simulink model "LDW_Real_Life_road_construction_cs"  (Simulink 7.6)
settings: Solver=ode5  SolverMode=SingleTasking  StartTime=0.0  StopTime=inf  FixedStep=1/100  RelTol=1e-3
PreLoadFcn:
  | check_NUTS_defined;
  | 
  | assignin('base',['model_closed_' bdroot],false);
  | 
  | if exist('LDW_mask_sfun.mexw64')==3
  | delete LDW_mask_sfun.mexw64;
  | end
  | 
  | if exist('LDW_mask_sfun.mexw32') ==3
  | delete LDW_mask_sfun.mexw32;
  | end
  | 
  | switch version('-release')
  | case {'2010a', '2010b', '2011a'}
  | addpath('../includes_LKAS/includes_LKAS_2010a');
  | case {'2011b', '2012a', '2012b'}
  | addpath('../includes_LKAS/includes_LKAS_2011b');
  | otherwise
  | addpath('../includes_LKAS/includes_LKAS_2011b');
  | end
InitFcn:
  | PreScanExperimentInitFcn(bdroot)
StopFcn:
  | PreScanExperimentStopFcn(bdroot)
undefined symbols (not defined in the file): DiscIntNeverNeededParam, InitImageOutputDir, x_ini_VRML, y_ini_VRML, z_ini_VRML
libraries: PreScanSimulinkLibrary x69, simulink x26, PreScanUsefulBlocks x25, simulink_extras x15, dspsnks4 x2, simulink_need_slupdate x1
(part 9 of 26)

== system LDW_Real_Life_road_construction_cs/VISUMatrixSignLights_HighwayOverhang_5 ==
  MatrixSignLights : Inport
  SubMuxVisuMatrixSignLights : Demux  Outputs=16
  conv_Orange_1 : Subsystem
  conv_Orange_10 : Subsystem
  conv_Orange_11 : Subsystem
  conv_Orange_12 : Subsystem
  conv_Orange_13 : Subsystem
  conv_Orange_14 : Subsystem
  conv_Orange_15 : Subsystem
  conv_Orange_16 : Subsystem
  conv_Orange_2 : Subsystem
  conv_Orange_3 : Subsystem
  conv_Orange_4 : Subsystem
  conv_Orange_5 : Subsystem
  conv_Orange_6 : Subsystem
  conv_Orange_7 : Subsystem
  conv_Orange_8 : Subsystem
  conv_Orange_9 : Subsystem
  visu_MatrixSignLights_1 : Subsystem  mask(ToSensorWorld){PortName=MatrixSign_17_Light_1, PortIndex=1865, PortWidth=16, TimeStep=1/100}
      mask prompts: PortName "Port Name [-]"; PortIndex "Index [-]"; PortWidth "Port Dimensions [-]"; TimeStep "Upload Period [s]"
      mask help: Sends signal(s) from Simulink to the Sensor World
      mask Help:
      | Sends signal(s) from Simulink to the Sensor World.
      | 
      | - Port Name [-]: specifies the name of the output terminal of the Simulink block.
      | - Index [-]: specifies the start position in the output vector sent to the Sensor World
      | - Port dimensions [-]: specifies the width (number of scalars) of the output port
      | - Upload period [s]: specifies the upload period (in seconds) of the port.
      mask Display: port_label('input', 1, PortName)
  visu_MatrixSignLights_10 : Subsystem  mask(ToSensorWorld){PortName=MatrixSign_19_Light_2, PortIndex=2009, PortWidth=16, TimeStep=1/100}
      mask prompts: PortName "Port Name [-]"; PortIndex "Index [-]"; PortWidth "Port Dimensions [-]"; TimeStep "Upload Period [s]"
      mask help: Sends signal(s) from Simulink to the Sensor World
      mask Help:
      | Sends signal(s) from Simulink to the Sensor World.
      | 
      | - Port Name [-]: specifies the name of the output terminal of the Simulink block.
      | - Index [-]: specifies the start position in the output vector sent to the Sensor World
      | - Port dimensions [-]: specifies the width (number of scalars) of the output port
      | - Upload period [s]: specifies the upload period (in seconds) of the port.
      mask Display: port_label('input', 1, PortName)
  visu_MatrixSignLights_11 : Subsystem  mask(ToSensorWorld){PortName=MatrixSign_19_Light_3, PortIndex=2025, PortWidth=16, TimeStep=1/100}
      mask prompts: PortName "Port Name [-]"; PortIndex "Index [-]"; PortWidth "Port Dimensions [-]"; TimeStep "Upload Period [s]"
      mask help: Sends signal(s) from Simulink to the Sensor World
      mask Help:
      | Sends signal(s) from Simulink to the Sensor World.
      | 
      | - Port Name [-]: specifies the name of the output terminal of the Simulink block.
      | - Index [-]: specifies the start position in the output vector sent to the Sensor World
      | - Port dimensions [-]: specifies the width (number of scalars) of the output port
      | - Upload period [s]: specifies the upload period (in seconds) of the port.
      mask Display: port_label('input', 1, PortName)
  visu_MatrixSignLights_12 : Subsystem  mask(ToSensorWorld){PortName=MatrixSign_19_Light_4, PortIndex=2041, PortWidth=16, TimeStep=1/100}
      mask prompts: PortName "Port Name [-]"; PortIndex "Index [-]"; PortWidth "Port Dimensions [-]"; TimeStep "Upload Period [s]"
      mask help: Sends signal(s) from Simulink to the Sensor World
      mask Help:
      | Sends signal(s) from Simulink to the Sensor World.
      | 
      | - Port Name [-]: specifies the name of the output terminal of the Simulink block.
      | - Index [-]: specifies the start position in the output vector sent to the Sensor World
      | - Port dimensions [-]: specifies the width (number of scalars) of the output port
      | - Upload period [s]: specifies the upload period (in seconds) of the port.
      mask Display: port_label('input', 1, PortName)
  visu_MatrixSignLights_13 : Subsystem  mask(ToSensorWorld){PortName=MatrixSign_20_Light_1, PortIndex=2057, PortWidth=16, TimeStep=1/100}
      mask prompts: PortName "Port Name [-]"; PortIndex "Index [-]"; PortWidth "Port Dimensions [-]"; TimeStep "Upload Period [s]"
      mask help: Sends signal(s) from Simulink to the Sensor World
      mask Help:
      | Sends signal(s) from Simulink to the Sensor World.
      | 
      | - Port Name [-]: specifies the name of the output terminal of the Simulink block.
      | - Index [-]: specifies the start position in the output vector sent to the Sensor World
      | - Port dimensions [-]: specifies the width (number of scalars) of the output port
      | - Upload period [s]: specifies the upload period (in seconds) of the port.
      mask Display: port_label('input', 1, PortName)
  visu_MatrixSignLights_14 : Subsystem  mask(ToSensorWorld){PortName=MatrixSign_20_Light_2, PortIndex=2073, PortWidth=16, TimeStep=1/100}
      mask prompts: PortName "Port Name [-]"; PortIndex "Index [-]"; PortWidth "Port Dimensions [-]"; TimeStep "Upload Period [s]"
      mask help: Sends signal(s) from Simulink to the Sensor World
      mask Help:
      | Sends signal(s) from Simulink to the Sensor World.
      | 
      | - Port Name [-]: specifies the name of the output terminal of the Simulink block.
      | - Index [-]: specifies the start position in the output vector sent to the Sensor World
      | - Port dimensions [-]: specifies the width (number of scalars) of the output port
      | - Upload period [s]: specifies the upload period (in seconds) of the port.
      mask Display: port_label('input', 1, PortName)
  visu_MatrixSignLights_15 : Subsystem  mask(ToSensorWorld){PortName=MatrixSign_20_Light_3, PortIndex=2089, PortWidth=16, TimeStep=1/100}
      mask prompts: PortName "Port Name [-]"; PortIndex "Index [-]"; PortWidth "Port Dimensions [-]"; TimeStep "Upload Period [s]"
      mask help: Sends signal(s) from Simulink to the Sensor World
      mask Help:
      | Sends signal(s) from Simulink to the Sensor World.
      | 
      | - Port Name [-]: specifies the name of the output terminal of the Simulink block.
      | - Index [-]: specifies the start position in the output vector sent to the Sensor World
      | - Port dimensions [-]: specifies the width (number of scalars) of the output port
      | - Upload period [s]: specifies the upload period (in seconds) of the port.
      mask Display: port_label('input', 1, PortName)
  visu_MatrixSignLights_16 : Subsystem  mask(ToSensorWorld){PortName=MatrixSign_20_Light_4, PortIndex=2105, PortWidth=16, TimeStep=1/100}
      mask prompts: PortName "Port Name [-]"; PortIndex "Index [-]"; PortWidth "Port Dimensions [-]"; TimeStep "Upload Period [s]"
      mask help: Sends signal(s) from Simulink to the Sensor World
      mask Help:
      | Sends signal(s) from Simulink to the Sensor World.
      | 
      | - Port Name [-]: specifies the name of the output terminal of the Simulink block.
      | - Index [-]: specifies the start position in the output vector sent to the Sensor World
      | - Port dimensions [-]: specifies the width (number of scalars) of the output port
      | - Upload period [s]: specifies the upload period (in seconds) of the port.
      mask Display: port_label('input', 1, PortName)
  visu_MatrixSignLights_2 : Subsystem  mask(ToSensorWorld){PortName=MatrixSign_17_Light_2, PortIndex=1881, PortWidth=16, TimeStep=1/100}
      mask prompts: PortName "Port Name [-]"; PortIndex "Index [-]"; PortWidth "Port Dimensions [-]"; TimeStep "Upload Period [s]"
      mask help: Sends signal(s) from Simulink to the Sensor World
      mask Help:
      | Sends signal(s) from Simulink to the Sensor World.
      | 
      | - Port Name [-]: specifies the name of the output terminal of the Simulink block.
      | - Index [-]: specifies the start position in the output vector sent to the Sensor World
      | - Port dimensions [-]: specifies the width (number of scalars) of the output port
      | - Upload period [s]: specifies the upload period (in seconds) of the port.
      mask Display: port_label('input', 1, PortName)
  visu_MatrixSignLights_3 : Subsystem  mask(ToSensorWorld){PortName=MatrixSign_17_Light_3, PortIndex=1897, PortWidth=16, TimeStep=1/100}
      mask prompts: PortName "Port Name [-]"; PortIndex "Index [-]"; PortWidth "Port Dimensions [-]"; TimeStep "Upload Period [s]"
      mask help: Sends signal(s) from Simulink to the Sensor World
      mask Help:
      | Sends signal(s) from Simulink to the Sensor World.
      | 
      | - Port Name [-]: specifies the name of the output terminal of the Simulink block.
      | - Index [-]: specifies the start position in the output vector sent to the Sensor World
      | - Port dimensions [-]: specifies the width (number of scalars) of the output port
      | - Upload period [s]: specifies the upload period (in seconds) of the port.
      mask Display: port_label('input', 1, PortName)
  visu_MatrixSignLights_4 : Subsystem  mask(ToSensorWorld){PortName=MatrixSign_17_Light_4, PortIndex=1913, PortWidth=16, TimeStep=1/100}
      mask prompts: PortName "Port Name [-]"; PortIndex "Index [-]"; PortWidth "Port Dimensions [-]"; TimeStep "Upload Period [s]"
      mask help: Sends signal(s) from Simulink to the Sensor World
      mask Help:
      | Sends signal(s) from Simulink to the Sensor World.
      | 
      | - Port Name [-]: specifies the name of the output terminal of the Simulink block.
      | - Index [-]: specifies the start position in the output vector sent to the Sensor World
      | - Port dimensions [-]: specifies the width (number of scalars) of the output port
      | - Upload period [s]: specifies the upload period (in seconds) of the port.
      mask Display: port_label('input', 1, PortName)
  visu_MatrixSignLights_5 : Subsystem  mask(ToSensorWorld){PortName=MatrixSign_18_Light_1, PortIndex=1929, PortWidth=16, TimeStep=1/100}
      mask prompts: PortName "Port Name [-]"; PortIndex "Index [-]"; PortWidth "Port Dimensions [-]"; TimeStep "Upload Period [s]"
      mask help: Sends signal(s) from Simulink to the Sensor World
      mask Help:
      | Sends signal(s) from Simulink to the Sensor World.
      | 
      | - Port Name [-]: specifies the name of the output terminal of the Simulink block.
      | - Index [-]: specifies the start position in the output vector sent to the Sensor World
      | - Port dimensions [-]: specifies the width (number of scalars) of the output port
      | - Upload period [s]: specifies the upload period (in seconds) of the port.
      mask Display: port_label('input', 1, PortName)
  visu_MatrixSignLights_6 : Subsystem  mask(ToSensorWorld){PortName=MatrixSign_18_Light_2, PortIndex=1945, PortWidth=16, TimeStep=1/100}
      mask prompts: PortName "Port Name [-]"; PortIndex "Index [-]"; PortWidth "Port Dimensions [-]"; TimeStep "Upload Period [s]"
      mask help: Sends signal(s) from Simulink to the Sensor World
      mask Help:
      | Sends signal(s) from Simulink to the Sensor World.
      | 
      | - Port Name [-]: specifies the name of the output terminal of the Simulink block.
      | - Index [-]: specifies the start position in the output vector sent to the Sensor World
      | - Port dimensions [-]: specifies the width (number of scalars) of the output port
      | - Upload period [s]: specifies the upload period (in seconds) of the port.
      mask Display: port_label('input', 1, PortName)
  visu_MatrixSignLights_7 : Subsystem  mask(ToSensorWorld){PortName=MatrixSign_18_Light_3, PortIndex=1961, PortWidth=16, TimeStep=1/100}
      mask prompts: PortName "Port Name [-]"; PortIndex "Index [-]"; PortWidth "Port Dimensions [-]"; TimeStep "Upload Period [s]"
      mask help: Sends signal(s) from Simulink to the Sensor World
      mask Help:
      | Sends signal(s) from Simulink to the Sensor World.
      | 
      | - Port Name [-]: specifies the name of the output terminal of the Simulink block.
      | - Index [-]: specifies the start position in the output vector sent to the Sensor World
      | - Port dimensions [-]: specifies the width (number of scalars) of the output port
      | - Upload period [s]: specifies the upload period (in seconds) of the port.
      mask Display: port_label('input', 1, PortName)
  visu_MatrixSignLights_8 : Subsystem  mask(ToSensorWorld){PortName=MatrixSign_18_Light_4, PortIndex=1977, PortWidth=16, TimeStep=1/100}
      mask prompts: PortName "Port Name [-]"; PortIndex "Index [-]"; PortWidth "Port Dimensions [-]"; TimeStep "Upload Period [s]"
      mask help: Sends signal(s) from Simulink to the Sensor World
      mask Help:
      | Sends signal(s) from Simulink to the Sensor World.
      | 
      | - Port Name [-]: specifies the name of the output terminal of the Simulink block.
      | - Index [-]: specifies the start position in the output vector sent to the Sensor World
      | - Port dimensions [-]: specifies the width (number of scalars) of the output port
      | - Upload period [s]: specifies the upload period (in seconds) of the port.
      mask Display: port_label('input', 1, PortName)
  visu_MatrixSignLights_9 : Subsystem  mask(ToSensorWorld){PortName=MatrixSign_19_Light_1, PortIndex=1993, PortWidth=16, TimeStep=1/100}
      mask prompts: PortName "Port Name [-]"; PortIndex "Index [-]"; PortWidth "Port Dimensions [-]"; TimeStep "Upload Period [s]"
      mask help: Sends signal(s) from Simulink to the Sensor World
      mask Help:
      | Sends signal(s) from Simulink to the Sensor World.
      | 
      | - Port Name [-]: specifies the name of the output terminal of the Simulink block.
      | - Index [-]: specifies the start position in the output vector sent to the Sensor World
      | - Port dimensions [-]: specifies the width (number of scalars) of the output port
      | - Upload period [s]: specifies the upload period (in seconds) of the port.
      mask Display: port_label('input', 1, PortName)
  wires:
  conv_Orange_1/1(RGBcolor) -> visu_MatrixSignLights_1/1(Y)
  MatrixSignLights/1 -> SubMuxVisuMatrixSignLights/1
  SubMuxVisuMatrixSignLights/1 -> conv_Orange_1/1(on_off)
  SubMuxVisuMatrixSignLights/2 -> conv_Orange_2/1(on_off)
  conv_Orange_2/1(RGBcolor) -> visu_MatrixSignLights_2/1(Y)
  SubMuxVisuMatrixSignLights/3 -> conv_Orange_3/1(on_off)
  conv_Orange_3/1(RGBcolor) -> visu_MatrixSignLights_3/1(Y)
  SubMuxVisuMatrixSignLights/4 -> conv_Orange_4/1(on_off)
  conv_Orange_4/1(RGBcolor) -> visu_MatrixSignLights_4/1(Y)
  SubMuxVisuMatrixSignLights/5 -> conv_Orange_5/1(on_off)
  conv_Orange_5/1(RGBcolor) -> visu_MatrixSignLights_5/1(Y)
  SubMuxVisuMatrixSignLights/6 -> conv_Orange_6/1(on_off)
  conv_Orange_6/1(RGBcolor) -> visu_MatrixSignLights_6/1(Y)
  SubMuxVisuMatrixSignLights/7 -> conv_Orange_7/1(on_off)
  conv_Orange_7/1(RGBcolor) -> visu_MatrixSignLights_7/1(Y)
  SubMuxVisuMatrixSignLights/8 -> conv_Orange_8/1(on_off)
  conv_Orange_8/1(RGBcolor) -> visu_MatrixSignLights_8/1(Y)
  SubMuxVisuMatrixSignLights/9 -> conv_Orange_9/1(on_off)
  conv_Orange_9/1(RGBcolor) -> visu_MatrixSignLights_9/1(Y)
  SubMuxVisuMatrixSignLights/10 -> conv_Orange_10/1(on_off)
  conv_Orange_10/1(RGBcolor) -> visu_MatrixSignLights_10/1(Y)
  SubMuxVisuMatrixSignLights/11 -> conv_Orange_11/1(on_off)
  conv_Orange_11/1(RGBcolor) -> visu_MatrixSignLights_11/1(Y)
  SubMuxVisuMatrixSignLights/12 -> conv_Orange_12/1(on_off)
  conv_Orange_12/1(RGBcolor) -> visu_MatrixSignLights_12/1(Y)
  SubMuxVisuMatrixSignLights/13 -> conv_Orange_13/1(on_off)
  conv_Orange_13/1(RGBcolor) -> visu_MatrixSignLights_13/1(Y)
  SubMuxVisuMatrixSignLights/14 -> conv_Orange_14/1(on_off)
  conv_Orange_14/1(RGBcolor) -> visu_MatrixSignLights_14/1(Y)
  SubMuxVisuMatrixSignLights/15 -> conv_Orange_15/1(on_off)
  conv_Orange_15/1(RGBcolor) -> visu_MatrixSignLights_15/1(Y)
  SubMuxVisuMatrixSignLights/16 -> conv_Orange_16/1(on_off)
  conv_Orange_16/1(RGBcolor) -> visu_MatrixSignLights_16/1(Y)

== system LDW_Real_Life_road_construction_cs/VISUMatrixSignLights_HighwayOverhang_6 ==
  MatrixSignLights : Inport
  SubMuxVisuMatrixSignLights : Demux  Outputs=16
  conv_Orange_1 : Subsystem
  conv_Orange_10 : Subsystem
  conv_Orange_11 : Subsystem
  conv_Orange_12 : Subsystem
  conv_Orange_13 : Subsystem
  conv_Orange_14 : Subsystem
  conv_Orange_15 : Subsystem
  conv_Orange_16 : Subsystem
  conv_Orange_2 : Subsystem
  conv_Orange_3 : Subsystem
  conv_Orange_4 : Subsystem
  conv_Orange_5 : Subsystem
  conv_Orange_6 : Subsystem
  conv_Orange_7 : Subsystem
  conv_Orange_8 : Subsystem
  conv_Orange_9 : Subsystem
  visu_MatrixSignLights_1 : Subsystem  mask(ToSensorWorld){PortName=MatrixSign_21_Light_1, PortIndex=2125, PortWidth=16, TimeStep=1/100}
      mask prompts: PortName "Port Name [-]"; PortIndex "Index [-]"; PortWidth "Port Dimensions [-]"; TimeStep "Upload Period [s]"
      mask help: Sends signal(s) from Simulink to the Sensor World
      mask Help:
      | Sends signal(s) from Simulink to the Sensor World.
      | 
      | - Port Name [-]: specifies the name of the output terminal of the Simulink block.
      | - Index [-]: specifies the start position in the output vector sent to the Sensor World
      | - Port dimensions [-]: specifies the width (number of scalars) of the output port
      | - Upload period [s]: specifies the upload period (in seconds) of the port.
      mask Display: port_label('input', 1, PortName)
  visu_MatrixSignLights_10 : Subsystem  mask(ToSensorWorld){PortName=MatrixSign_23_Light_2, PortIndex=2269, PortWidth=16, TimeStep=1/100}
      mask prompts: PortName "Port Name [-]"; PortIndex "Index [-]"; PortWidth "Port Dimensions [-]"; TimeStep "Upload Period [s]"
      mask help: Sends signal(s) from Simulink to the Sensor World
      mask Help:
      | Sends signal(s) from Simulink to the Sensor World.
      | 
      | - Port Name [-]: specifies the name of the output terminal of the Simulink block.
      | - Index [-]: specifies the start position in the output vector sent to the Sensor World
      | - Port dimensions [-]: specifies the width (number of scalars) of the output port
      | - Upload period [s]: specifies the upload period (in seconds) of the port.
      mask Display: port_label('input', 1, PortName)
  visu_MatrixSignLights_11 : Subsystem  mask(ToSensorWorld){PortName=MatrixSign_23_Light_3, PortIndex=2285, PortWidth=16, TimeStep=1/100}
      mask prompts: PortName "Port Name [-]"; PortIndex "Index [-]"; PortWidth "Port Dimensions [-]"; TimeStep "Upload Period [s]"
      mask help: Sends signal(s) from Simulink to the Sensor World
      mask Help:
      | Sends signal(s) from Simulink to the Sensor World.
      | 
      | - Port Name [-]: specifies the name of the output terminal of the Simulink block.
      | - Index [-]: specifies the start position in the output vector sent to the Sensor World
      | - Port dimensions [-]: specifies the width (number of scalars) of the output port
      | - Upload period [s]: specifies the upload period (in seconds) of the port.
      mask Display: port_label('input', 1, PortName)
  visu_MatrixSignLights_12 : Subsystem  mask(ToSensorWorld){PortName=MatrixSign_23_Light_4, PortIndex=2301, PortWidth=16, TimeStep=1/100}
      mask prompts: PortName "Port Name [-]"; PortIndex "Index [-]"; PortWidth "Port Dimensions [-]"; TimeStep "Upload Period [s]"
      mask help: Sends signal(s) from Simulink to the Sensor World
      mask Help:
      | Sends signal(s) from Simulink to the Sensor World.
      | 
      | - Port Name [-]: specifies the name of the output terminal of the Simulink block.
      | - Index [-]: specifies the start position in the output vector sent to the Sensor World
      | - Port dimensions [-]: specifies the width (number of scalars) of the output port
      | - Upload period [s]: specifies the upload period (in seconds) of the port.
      mask Display: port_label('input', 1, PortName)
  visu_MatrixSignLights_13 : Subsystem  mask(ToSensorWorld){PortName=MatrixSign_24_Light_1, PortIndex=2317, PortWidth=16, TimeStep=1/100}
      mask prompts: PortName "Port Name [-]"; PortIndex "Index [-]"; PortWidth "Port Dimensions [-]"; TimeStep "Upload Period [s]"
      mask help: Sends signal(s) from Simulink to the Sensor World
      mask Help:
      | Sends signal(s) from Simulink to the Sensor World.
      | 
      | - Port Name [-]: specifies the name of the output terminal of the Simulink block.
      | - Index [-]: specifies the start position in the output vector sent to the Sensor World
      | - Port dimensions [-]: specifies the width (number of scalars) of the output port
      | - Upload period [s]: specifies the upload period (in seconds) of the port.
      mask Display: port_label('input', 1, PortName)
  visu_MatrixSignLights_14 : Subsystem  mask(ToSensorWorld){PortName=MatrixSign_24_Light_2, PortIndex=2333, PortWidth=16, TimeStep=1/100}
      mask prompts: PortName "Port Name [-]"; PortIndex "Index [-]"; PortWidth "Port Dimensions [-]"; TimeStep "Upload Period [s]"
      mask help: Sends signal(s) from Simulink to the Sensor World
      mask Help:
      | Sends signal(s) from Simulink to the Sensor World.
      | 
      | - Port Name [-]: specifies the name of the output terminal of the Simulink block.
      | - Index [-]: specifies the start position in the output vector sent to the Sensor World
      | - Port dimensions [-]: specifies the width (number of scalars) of the output port
      | - Upload period [s]: specifies the upload period (in seconds) of the port.
      mask Display: port_label('input', 1, PortName)
  visu_MatrixSignLights_15 : Subsystem  mask(ToSensorWorld){PortName=MatrixSign_24_Light_3, PortIndex=2349, PortWidth=16, TimeStep=1/100}
      mask prompts: PortName "Port Name [-]"; PortIndex "Index [-]"; PortWidth "Port Dimensions [-]"; TimeStep "Upload Period [s]"
      mask help: Sends signal(s) from Simulink to the Sensor World
      mask Help:
      | Sends signal(s) from Simulink to the Sensor World.
      | 
      | - Port Name [-]: specifies the name of the output terminal of the Simulink block.
      | - Index [-]: specifies the start position in the output vector sent to the Sensor World
      | - Port dimensions [-]: specifies the width (number of scalars) of the output port
      | - Upload period [s]: specifies the upload period (in seconds) of the port.
      mask Display: port_label('input', 1, PortName)
  visu_MatrixSignLights_16 : Subsystem  mask(ToSensorWorld){PortName=MatrixSign_24_Light_4, PortIndex=2365, PortWidth=16, TimeStep=1/100}
      mask prompts: PortName "Port Name [-]"; PortIndex "Index [-]"; PortWidth "Port Dimensions [-]"; TimeStep "Upload Period [s]"
      mask help: Sends signal(s) from Simulink to the Sensor World
      mask Help:
      | Sends signal(s) from Simulink to the Sensor World.
      | 
      | - Port Name [-]: specifies the name of the output terminal of the Simulink block.
      | - Index [-]: specifies the start position in the output vector sent to the Sensor World
      | - Port dimensions [-]: specifies the width (number of scalars) of the output port
      | - Upload period [s]: specifies the upload period (in seconds) of the port.
      mask Display: port_label('input', 1, PortName)
  visu_MatrixSignLights_2 : Subsystem  mask(ToSensorWorld){PortName=MatrixSign_21_Light_2, PortIndex=2141, PortWidth=16, TimeStep=1/100}
      mask prompts: PortName "Port Name [-]"; PortIndex "Index [-]"; PortWidth "Port Dimensions [-]"; TimeStep "Upload Period [s]"
      mask help: Sends signal(s) from Simulink to the Sensor World
      mask Help:
      | Sends signal(s) from Simulink to the Sensor World.
      | 
      | - Port Name [-]: specifies the name of the output terminal of the Simulink block.
      | - Index [-]: specifies the start position in the output vector sent to the Sensor World
      | - Port dimensions [-]: specifies the width (number of scalars) of the output port
      | - Upload period [s]: specifies the upload period (in seconds) of the port.
      mask Display: port_label('input', 1, PortName)
  visu_MatrixSignLights_3 : Subsystem  mask(ToSensorWorld){PortName=MatrixSign_21_Light_3, PortIndex=2157, PortWidth=16, TimeStep=1/100}
      mask prompts: PortName "Port Name [-]"; PortIndex "Index [-]"; PortWidth "Port Dimensions [-]"; TimeStep "Upload Period [s]"
      mask help: Sends signal(s) from Simulink to the Sensor World
      mask Help:
      | Sends signal(s) from Simulink to the Sensor World.
      | 
      | - Port Name [-]: specifies the name of the output terminal of the Simulink block.
      | - Index [-]: specifies the start position in the output vector sent to the Sensor World
      | - Port dimensions [-]: specifies the width (number of scalars) of the output port
      | - Upload period [s]: specifies the upload period (in seconds) of the port.
      mask Display: port_label('input', 1, PortName)
  visu_MatrixSignLights_4 : Subsystem  mask(ToSensorWorld){PortName=MatrixSign_21_Light_4, PortIndex=2173, PortWidth=16, TimeStep=1/100}
      mask prompts: PortName "Port Name [-]"; PortIndex "Index [-]"; PortWidth "Port Dimensions [-]"; TimeStep "Upload Period [s]"
      mask help: Sends signal(s) from Simulink to the Sensor World
      mask Help:
      | Sends signal(s) from Simulink to the Sensor World.
      | 
      | - Port Name [-]: specifies the name of the output terminal of the Simulink block.
      | - Index [-]: specifies the start position in the output vector sent to the Sensor World
      | - Port dimensions [-]: specifies the width (number of scalars) of the output port
      | - Upload period [s]: specifies the upload period (in seconds) of the port.
      mask Display: port_label('input', 1, PortName)
  visu_MatrixSignLights_5 : Subsystem  mask(ToSensorWorld){PortName=MatrixSign_22_Light_1, PortIndex=2189, PortWidth=16, TimeStep=1/100}
      mask prompts: PortName "Port Name [-]"; PortIndex "Index [-]"; PortWidth "Port Dimensions [-]"; TimeStep "Upload Period [s]"
      mask help: Sends signal(s) from Simulink to the Sensor World
      mask Help:
      | Sends signal(s) from Simulink to the Sensor World.
      | 
      | - Port Name [-]: specifies the name of the output terminal of the Simulink block.
      | - Index [-]: specifies the start position in the output vector sent to the Sensor World
      | - Port dimensions [-]: specifies the width (number of scalars) of the output port
      | - Upload period [s]: specifies the upload period (in seconds) of the port.
      mask Display: port_label('input', 1, PortName)
  visu_MatrixSignLights_6 : Subsystem  mask(ToSensorWorld){PortName=MatrixSign_22_Light_2, PortIndex=2205, PortWidth=16, TimeStep=1/100}
      mask prompts: PortName "Port Name [-]"; PortIndex "Index [-]"; PortWidth "Port Dimensions [-]"; TimeStep "Upload Period [s]"
      mask help: Sends signal(s) from Simulink to the Sensor World
      mask Help:
      | Sends signal(s) from Simulink to the Sensor World.
      | 
      | - Port Name [-]: specifies the name of the output terminal of the Simulink block.
      | - Index [-]: specifies the start position in the output vector sent to the Sensor World
      | - Port dimensions [-]: specifies the width (number of scalars) of the output port
      | - Upload period [s]: specifies the upload period (in seconds) of the port.
      mask Display: port_label('input', 1, PortName)
  visu_MatrixSignLights_7 : Subsystem  mask(ToSensorWorld){PortName=MatrixSign_22_Light_3, PortIndex=2221, PortWidth=16, TimeStep=1/100}
      mask prompts: PortName "Port Name [-]"; PortIndex "Index [-]"; PortWidth "Port Dimensions [-]"; TimeStep "Upload Period [s]"
      mask help: Sends signal(s) from Simulink to the Sensor World
      mask Help:
      | Sends signal(s) from Simulink to the Sensor World.
      | 
      | - Port Name [-]: specifies the name of the output terminal of the Simulink block.
      | - Index [-]: specifies the start position in the output vector sent to the Sensor World
      | - Port dimensions [-]: specifies the width (number of scalars) of the output port
      | - Upload period [s]: specifies the upload period (in seconds) of the port.
      mask Display: port_label('input', 1, PortName)
  visu_MatrixSignLights_8 : Subsystem  mask(ToSensorWorld){PortName=MatrixSign_22_Light_4, PortIndex=2237, PortWidth=16, TimeStep=1/100}
      mask prompts: PortName "Port Name [-]"; PortIndex "Index [-]"; PortWidth "Port Dimensions [-]"; TimeStep "Upload Period [s]"
      mask help: Sends signal(s) from Simulink to the Sensor World
      mask Help:
      | Sends signal(s) from Simulink to the Sensor World.
      | 
      | - Port Name [-]: specifies the name of the output terminal of the Simulink block.
      | - Index [-]: specifies the start position in the output vector sent to the Sensor World
      | - Port dimensions [-]: specifies the width (number of scalars) of the output port
      | - Upload period [s]: specifies the upload period (in seconds) of the port.
      mask Display: port_label('input', 1, PortName)
  visu_MatrixSignLights_9 : Subsystem  mask(ToSensorWorld){PortName=MatrixSign_23_Light_1, PortIndex=2253, PortWidth=16, TimeStep=1/100}
      mask prompts: PortName "Port Name [-]"; PortIndex "Index [-]"; PortWidth "Port Dimensions [-]"; TimeStep "Upload Period [s]"
      mask help: Sends signal(s) from Simulink to the Sensor World
      mask Help:
      | Sends signal(s) from Simulink to the Sensor World.
      | 
      | - Port Name [-]: specifies the name of the output terminal of the Simulink block.
      | - Index [-]: specifies the start position in the output vector sent to the Sensor World
      | - Port dimensions [-]: specifies the width (number of scalars) of the output port
      | - Upload period [s]: specifies the upload period (in seconds) of the port.
      mask Display: port_label('input', 1, PortName)
  wires:
  conv_Orange_1/1(RGBcolor) -> visu_MatrixSignLights_1/1(Y)
  MatrixSignLights/1 -> SubMuxVisuMatrixSignLights/1
  SubMuxVisuMatrixSignLights/1 -> conv_Orange_1/1(on_off)
  SubMuxVisuMatrixSignLights/2 -> conv_Orange_2/1(on_off)
  conv_Orange_2/1(RGBcolor) -> visu_MatrixSignLights_2/1(Y)
  SubMuxVisuMatrixSignLights/3 -> conv_Orange_3/1(on_off)
  conv_Orange_3/1(RGBcolor) -> visu_MatrixSignLights_3/1(Y)
  SubMuxVisuMatrixSignLights/4 -> conv_Orange_4/1(on_off)
  conv_Orange_4/1(RGBcolor) -> visu_MatrixSignLights_4/1(Y)
  SubMuxVisuMatrixSignLights/5 -> conv_Orange_5/1(on_off)
  conv_Orange_5/1(RGBcolor) -> visu_MatrixSignLights_5/1(Y)
  SubMuxVisuMatrixSignLights/6 -> conv_Orange_6/1(on_off)
  conv_Orange_6/1(RGBcolor) -> visu_MatrixSignLights_6/1(Y)
  SubMuxVisuMatrixSignLights/7 -> conv_Orange_7/1(on_off)
  conv_Orange_7/1(RGBcolor) -> visu_MatrixSignLights_7/1(Y)
  SubMuxVisuMatrixSignLights/8 -> conv_Orange_8/1(on_off)
  conv_Orange_8/1(RGBcolor) -> visu_MatrixSignLights_8/1(Y)
  SubMuxVisuMatrixSignLights/9 -> conv_Orange_9/1(on_off)
  conv_Orange_9/1(RGBcolor) -> visu_MatrixSignLights_9/1(Y)
  SubMuxVisuMatrixSignLights/10 -> conv_Orange_10/1(on_off)
  conv_Orange_10/1(RGBcolor) -> visu_MatrixSignLights_10/1(Y)
  SubMuxVisuMatrixSignLights/11 -> conv_Orange_11/1(on_off)
  conv_Orange_11/1(RGBcolor) -> visu_MatrixSignLights_11/1(Y)
  SubMuxVisuMatrixSignLights/12 -> conv_Orange_12/1(on_off)
  conv_Orange_12/1(RGBcolor) -> visu_MatrixSignLights_12/1(Y)
  SubMuxVisuMatrixSignLights/13 -> conv_Orange_13/1(on_off)
  conv_Orange_13/1(RGBcolor) -> visu_MatrixSignLights_13/1(Y)
  SubMuxVisuMatrixSignLights/14 -> conv_Orange_14/1(on_off)
  conv_Orange_14/1(RGBcolor) -> visu_MatrixSignLights_14/1(Y)
  SubMuxVisuMatrixSignLights/15 -> conv_Orange_15/1(on_off)
  conv_Orange_15/1(RGBcolor) -> visu_MatrixSignLights_15/1(Y)
  SubMuxVisuMatrixSignLights/16 -> conv_Orange_16/1(on_off)
  conv_Orange_16/1(RGBcolor) -> visu_MatrixSignLights_16/1(Y)

== system LDW_Real_Life_road_construction_cs/VISUMatrixSign_HighwayOverhang_1 ==
  MatrixSigns : Inport
  SubMuxVisuMatrixSign : Demux
  visu_MatrixSign_1 : Subsystem  mask(ToSensorWorld){PortName=MatrixSign_1, PortIndex=821, PortWidth=1, TimeStep=1/100}
      mask prompts: PortName "Port Name [-]"; PortIndex "Index [-]"; PortWidth "Port Dimensions [-]"; TimeStep "Upload Period [s]"
      mask help: Sends signal(s) from Simulink to the Sensor World
      mask Help:
      | Sends signal(s) from Simulink to the Sensor World.
      | 
      | - Port Name [-]: specifies the name of the output terminal of the Simulink block.
      | - Index [-]: specifies the start position in the output vector sent to the Sensor World
      | - Port dimensions [-]: specifies the width (number of scalars) of the output port
      | - Upload period [s]: specifies the upload period (in seconds) of the port.
      mask Display: port_label('input', 1, PortName)
  visu_MatrixSign_2 : Subsystem  mask(ToSensorWorld){PortName=MatrixSign_2, PortIndex=822, PortWidth=1, TimeStep=1/100}
      mask prompts: PortName "Port Name [-]"; PortIndex "Index [-]"; PortWidth "Port Dimensions [-]"; TimeStep "Upload Period [s]"
      mask help: Sends signal(s) from Simulink to the Sensor World
      mask Help:
      | Sends signal(s) from Simulink to the Sensor World.
      | 
      | - Port Name [-]: specifies the name of the output terminal of the Simulink block.
      | - Index [-]: specifies the start position in the output vector sent to the Sensor World
      | - Port dimensions [-]: specifies the width (number of scalars) of the output port
      | - Upload period [s]: specifies the upload period (in seconds) of the port.
      mask Display: port_label('input', 1, PortName)
  visu_MatrixSign_3 : Subsystem  mask(ToSensorWorld){PortName=MatrixSign_3, PortIndex=823, PortWidth=1, TimeStep=1/100}
      mask prompts: PortName "Port Name [-]"; PortIndex "Index [-]"; PortWidth "Port Dimensions [-]"; TimeStep "Upload Period [s]"
      mask help: Sends signal(s) from Simulink to the Sensor World
      mask Help:
      | Sends signal(s) from Simulink to the Sensor World.
      | 
      | - Port Name [-]: specifies the name of the output terminal of the Simulink block.
      | - Index [-]: specifies the start position in the output vector sent to the Sensor World
      | - Port dimensions [-]: specifies the width (number of scalars) of the output port
      | - Upload period [s]: specifies the upload period (in seconds) of the port.
      mask Display: port_label('input', 1, PortName)
  visu_MatrixSign_4 : Subsystem  mask(ToSensorWorld){PortName=MatrixSign_4, PortIndex=824, PortWidth=1, TimeStep=1/100}
      mask prompts: PortName "Port Name [-]"; PortIndex "Index [-]"; PortWidth "Port Dimensions [-]"; TimeStep "Upload Period [s]"
      mask help: Sends signal(s) from Simulink to the Sensor World
      mask Help:
      | Sends signal(s) from Simulink to the Sensor World.
      | 
      | - Port Name [-]: specifies the name of the output terminal of the Simulink block.
      | - Index [-]: specifies the start position in the output vector sent to the Sensor World
      | - Port dimensions [-]: specifies the width (number of scalars) of the output port
      | - Upload period [s]: specifies the upload period (in seconds) of the port.
      mask Display: port_label('input', 1, PortName)
  wires:
  SubMuxVisuMatrixSign/1 -> visu_MatrixSign_1/1(Y)
  MatrixSigns/1 -> SubMuxVisuMatrixSign/1
  SubMuxVisuMatrixSign/2 -> visu_MatrixSign_2/1(Y)
  SubMuxVisuMatrixSign/3 -> visu_MatrixSign_3/1(Y)
  SubMuxVisuMatrixSign/4 -> visu_MatrixSign_4/1(Y)

== system LDW_Real_Life_road_construction_cs/VISUMatrixSign_HighwayOverhang_2 ==
  MatrixSigns : Inport
  SubMuxVisuMatrixSign : Demux
  visu_MatrixSign_1 : Subsystem  mask(ToSensorWorld){PortName=MatrixSign_5, PortIndex=1081, PortWidth=1, TimeStep=1/100}
      mask prompts: PortName "Port Name [-]"; PortIndex "Index [-]"; PortWidth "Port Dimensions [-]"; TimeStep "Upload Period [s]"
      mask help: Sends signal(s) from Simulink to the Sensor World
      mask Help:
      | Sends signal(s) from Simulink to the Sensor World.
      | 
      | - Port Name [-]: specifies the name of the output terminal of the Simulink block.
      | - Index [-]: specifies the start position in the output vector sent to the Sensor World
      | - Port dimensions [-]: specifies the width (number of scalars) of the output port
      | - Upload period [s]: specifies the upload period (in seconds) of the port.
      mask Display: port_label('input', 1, PortName)
  visu_MatrixSign_2 : Subsystem  mask(ToSensorWorld){PortName=MatrixSign_6, PortIndex=1082, PortWidth=1, TimeStep=1/100}
      mask prompts: PortName "Port Name [-]"; PortIndex "Index [-]"; PortWidth "Port Dimensions [-]"; TimeStep "Upload Period [s]"
      mask help: Sends signal(s) from Simulink to the Sensor World
      mask Help:
      | Sends signal(s) from Simulink to the Sensor World.
      | 
      | - Port Name [-]: specifies the name of the output terminal of the Simulink block.
      | - Index [-]: specifies the start position in the output vector sent to the Sensor World
      | - Port dimensions [-]: specifies the width (number of scalars) of the output port
      | - Upload period [s]: specifies the upload period (in seconds) of the port.
      mask Display: port_label('input', 1, PortName)
  visu_MatrixSign_3 : Subsystem  mask(ToSensorWorld){PortName=MatrixSign_7, PortIndex=1083, PortWidth=1, TimeStep=1/100}
      mask prompts: PortName "Port Name [-]"; PortIndex "Index [-]"; PortWidth "Port Dimensions [-]"; TimeStep "Upload Period [s]"
      mask help: Sends signal(s) from Simulink to the Sensor World
      mask Help:
      | Sends signal(s) from Simulink to the Sensor World.
      | 
      | - Port Name [-]: specifies the name of the output terminal of the Simulink block.
      | - Index [-]: specifies the start position in the output vector sent to the Sensor World
      | - Port dimensions [-]: specifies the width (number of scalars) of the output port
      | - Upload period [s]: specifies the upload period (in seconds) of the port.
      mask Display: port_label('input', 1, PortName)
  visu_MatrixSign_4 : Subsystem  mask(ToSensorWorld){PortName=MatrixSign_8, PortIndex=1084, PortWidth=1, TimeStep=1/100}
      mask prompts: PortName "Port Name [-]"; PortIndex "Index [-]"; PortWidth "Port Dimensions [-]"; TimeStep "Upload Period [s]"
      mask help: Sends signal(s) from Simulink to the Sensor World
      mask Help:
      | Sends signal(s) from Simulink to the Sensor World.
      | 
      | - Port Name [-]: specifies the name of the output terminal of the Simulink block.
      | - Index [-]: specifies the start position in the output vector sent to the Sensor World
      | - Port dimensions [-]: specifies the width (number of scalars) of the output port
      | - Upload period [s]: specifies the upload period (in seconds) of the port.
      mask Display: port_label('input', 1, PortName)
  wires:
  SubMuxVisuMatrixSign/1 -> visu_MatrixSign_1/1(Y)
  MatrixSigns/1 -> SubMuxVisuMatrixSign/1
  SubMuxVisuMatrixSign/2 -> visu_MatrixSign_2/1(Y)
  SubMuxVisuMatrixSign/3 -> visu_MatrixSign_3/1(Y)
  SubMuxVisuMatrixSign/4 -> visu_MatrixSign_4/1(Y)

== system LDW_Real_Life_road_construction_cs/VISUMatrixSign_HighwayOverhang_3 ==
  MatrixSigns : Inport
  SubMuxVisuMatrixSign : Demux
  visu_MatrixSign_1 : Subsystem  mask(ToSensorWorld){PortName=MatrixSign_9, PortIndex=1341, PortWidth=1, TimeStep=1/100}
      mask prompts: PortName "Port Name [-]"; PortIndex "Index [-]"; PortWidth "Port Dimensions [-]"; TimeStep "Upload Period [s]"
      mask help: Sends signal(s) from Simulink to the Sensor World
      mask Help:
      | Sends signal(s) from Simulink to the Sensor World.
      | 
      | - Port Name [-]: specifies the name of the output terminal of the Simulink block.
      | - Index [-]: specifies the start position in the output vector sent to the Sensor World
      | - Port dimensions [-]: specifies the width (number of scalars) of the output port
      | - Upload period [s]: specifies the upload period (in seconds) of the port.
      mask Display: port_label('input', 1, PortName)
  visu_MatrixSign_2 : Subsystem  mask(ToSensorWorld){PortName=MatrixSign_10, PortIndex=1342, PortWidth=1, TimeStep=1/100}
      mask prompts: PortName "Port Name [-]"; PortIndex "Index [-]"; PortWidth "Port Dimensions [-]"; TimeStep "Upload Period [s]"
      mask help: Sends signal(s) from Simulink to the Sensor World
      mask Help:
      | Sends signal(s) from Simulink to the Sensor World.
      | 
      | - Port Name [-]: specifies the name of the output terminal of the Simulink block.
      | - Index [-]: specifies the start position in the output vector sent to the Sensor World
      | - Port dimensions [-]: specifies the width (number of scalars) of the output port
      | - Upload period [s]: specifies the upload period (in seconds) of the port.
      mask Display: port_label('input', 1, PortName)
  visu_MatrixSign_3 : Subsystem  mask(ToSensorWorld){PortName=MatrixSign_11, PortIndex=1343, PortWidth=1, TimeStep=1/100}
      mask prompts: PortName "Port Name [-]"; PortIndex "Index [-]"; PortWidth "Port Dimensions [-]"; TimeStep "Upload Period [s]"
      mask help: Sends signal(s) from Simulink to the Sensor World
      mask Help:
      | Sends signal(s) from Simulink to the Sensor World.
      | 
      | - Port Name [-]: specifies the name of the output terminal of the Simulink block.
      | - Index [-]: specifies the start position in the output vector sent to the Sensor World
      | - Port dimensions [-]: specifies the width (number of scalars) of the output port
      | - Upload period [s]: specifies the upload period (in seconds) of the port.
      mask Display: port_label('input', 1, PortName)
  visu_MatrixSign_4 : Subsystem  mask(ToSensorWorld){PortName=MatrixSign_12, PortIndex=1344, PortWidth=1, TimeStep=1/100}
      mask prompts: PortName "Port Name [-]"; PortIndex "Index [-]"; PortWidth "Port Dimensions [-]"; TimeStep "Upload Period [s]"
      mask help: Sends signal(s) from Simulink to the Sensor World
      mask Help:
      | Sends signal(s) from Simulink to the Sensor World.
      | 
      | - Port Name [-]: specifies the name of the output terminal of the Simulink block.
      | - Index [-]: specifies the start position in the output vector sent to the Sensor World
      | - Port dimensions [-]: specifies the width (number of scalars) of the output port
      | - Upload period [s]: specifies the upload period (in seconds) of the port.
      mask Display: port_label('input', 1, PortName)
  wires:
  SubMuxVisuMatrixSign/1 -> visu_MatrixSign_1/1(Y)
  MatrixSigns/1 -> SubMuxVisuMatrixSign/1
  SubMuxVisuMatrixSign/2 -> visu_MatrixSign_2/1(Y)
  SubMuxVisuMatrixSign/3 -> visu_MatrixSign_3/1(Y)
  SubMuxVisuMatrixSign/4 -> visu_MatrixSign_4/1(Y)

== system LDW_Real_Life_road_construction_cs/VISUMatrixSign_HighwayOverhang_4 ==
  MatrixSigns : Inport
  SubMuxVisuMatrixSign : Demux
  visu_MatrixSign_1 : Subsystem  mask(ToSensorWorld){PortName=MatrixSign_13, PortIndex=1601, PortWidth=1, TimeStep=1/100}
      mask prompts: PortName "Port Name [-]"; PortIndex "Index [-]"; PortWidth "Port Dimensions [-]"; TimeStep "Upload Period [s]"
      mask help: Sends signal(s) from Simulink to the Sensor World
      mask Help:
      | Sends signal(s) from Simulink to the Sensor World.
      | 
      | - Port Name [-]: specifies the name of the output terminal of the Simulink block.
      | - Index [-]: specifies the start position in the output vector sent to the Sensor World
      | - Port dimensions [-]: specifies the width (number of scalars) of the output port
      | - Upload period [s]: specifies the upload period (in seconds) of the port.
      mask Display: port_label('input', 1, PortName)
  visu_MatrixSign_2 : Subsystem  mask(ToSensorWorld){PortName=MatrixSign_14, PortIndex=1602, PortWidth=1, TimeStep=1/100}
      mask prompts: PortName "Port Name [-]"; PortIndex "Index [-]"; PortWidth "Port Dimensions [-]"; TimeStep "Upload Period [s]"
      mask help: Sends signal(s) from Simulink to the Sensor World
      mask Help:
      | Sends signal(s) from Simulink to the Sensor World.
      | 
      | - Port Name [-]: specifies the name of the output terminal of the Simulink block.
      | - Index [-]: specifies the start position in the output vector sent to the Sensor World
      | - Port dimensions [-]: specifies the width (number of scalars) of the output port
      | - Upload period [s]: specifies the upload period (in seconds) of the port.
      mask Display: port_label('input', 1, PortName)
  visu_MatrixSign_3 : Subsystem  mask(ToSensorWorld){PortName=MatrixSign_15, PortIndex=1603, PortWidth=1, TimeStep=1/100}
      mask prompts: PortName "Port Name [-]"; PortIndex "Index [-]"; PortWidth "Port Dimensions [-]"; TimeStep "Upload Period [s]"
      mask help: Sends signal(s) from Simulink to the Sensor World
      mask Help:
      | Sends signal(s) from Simulink to the Sensor World.
      | 
      | - Port Name [-]: specifies the name of the output terminal of the Simulink block.
      | - Index [-]: specifies the start position in the output vector sent to the Sensor World
      | - Port dimensions [-]: specifies the width (number of scalars) of the output port
      | - Upload period [s]: specifies the upload period (in seconds) of the port.
      mask Display: port_label('input', 1, PortName)
  visu_MatrixSign_4 : Subsystem  mask(ToSensorWorld){PortName=MatrixSign_16, PortIndex=1604, PortWidth=1, TimeStep=1/100}
      mask prompts: PortName "Port Name [-]"; PortIndex "Index [-]"; PortWidth "Port Dimensions [-]"; TimeStep "Upload Period [s]"
      mask help: Sends signal(s) from Simulink to the Sensor World
      mask Help:
      | Sends signal(s) from Simulink to the Sensor World.
      | 
      | - Port Name [-]: specifies the name of the output terminal of the Simulink block.
      | - Index [-]: specifies the start position in the output vector sent to the Sensor World
      | - Port dimensions [-]: specifies the width (number of scalars) of the output port
      | - Upload period [s]: specifies the upload period (in seconds) of the port.
      mask Display: port_label('input', 1, PortName)
  wires:
  SubMuxVisuMatrixSign/1 -> visu_MatrixSign_1/1(Y)
  MatrixSigns/1 -> SubMuxVisuMatrixSign/1
  SubMuxVisuMatrixSign/2 -> visu_MatrixSign_2/1(Y)
  SubMuxVisuMatrixSign/3 -> visu_MatrixSign_3/1(Y)
  SubMuxVisuMatrixSign/4 -> visu_MatrixSign_4/1(Y)
